simulink model "TosAeb"  (R2021a)
settings: Solver=FixedStepDiscrete  StartTime=0.0  StopTime=inf  FixedStep=0.01  RelTol=1e-3
undefined symbols (not defined in the file or in its folder): ACCModSts, AccSts1, AEB_ObjectMovingOrientation, AEB_ObjectMovingState, CN_BTCActive, CN_CurrentGearR, CN_CurrentGearR_DHT, CN_PTCActive, CN_VDCActive, CN_VSC_EspOff, CN_VSC_FCW_AEB_Error, CN_VSC_FCW_AEB_NoDispay, CN_VSC_FCW_AEB_PerfmDgdtn, CN_VSC_FCW_AEB_TempUnAvlbl, CN_VSC_VehStandstill, CNaccelAvoidMax, CNaccelAvoidMin, CNbrakePedalPress, CNcomfortStrLeftAccelMax, CNcomfortStrLeftAccelMin, CNcomfortStrRightAccelMax, CNcomfortStrRightAccelMin, CNDrvDoorOpen, CNDrvModeEco, CNDrvModeNorm, CNDrvModeOther, CNDrvModeSport, CNDrvSBRreminder, CNNull, CNobjInvalidID, CNPassDoorOpen, CNPassSBRreminder, CNPEBSAccelNegInf, CNPEBSAccelNull, CNPEBSTimeInf, CNPEBSTimeNull, CNSTOPMAX, CNSTOPNULL, CNsubjWidth, CNSwtOFF, CNSwtON, CNTTCMAX, CNTTCNULL, CNVehConfigAEBCycNone, CNVehConfigAEBNone, CNVehConfigAEBPedNone, CNVehConfigFCWNone, CNVehConfigJANone, CollisionSeverity, FALSE, FCWSnvty, JACycObjCalcInfoDefaultParams, JAPedObjCalcInfoDefaultParams, JAVehObjCalcInfoDefaultParams, latCycObjCalcInfoDefaultParams, latPedObjCalcInfoDefaultParams, longObjCalcInfoDefaultParams, ModSts, movingStatus, ObjectType ... (+1112)
libraries: simulink x3031, AEBSFunctionLib x50, Ta_Library x33, AEBSStateMachineLib x23, Mylibrary x12, simulink_extras x7, ObjSelect_lib x3, dspstat3 x2
(part 26 of 102)

== system TosAeb/FcwAebJa_Step_sys/ALG_AEBSystem/ALG_AEBController/AEB_FuncImpManagementApp/FuncBlockTimeCalc/tBlockTimeBrkReadyCalc/blockTimeTriggerCondCalc ==
  AEBModStsFuncImpArb : Inport  OutDataTypeStr=Bus: BusAEBModStsFuncImpArb
  Bus Selector : BusSelector  OutputSignals=brkReadyFuncImpArb.JA_pedPrefillFuncImpArb,brkReadyFuncImpArb.prefillFuncImpArb,brkReadyFuncImpArb.pedPrefillFuncImpArb,brkReadyFuncImpArb.cycPrefillFuncImpArb
  Bus Selector1 : BusSelector  OutputSignals=brkReadyFuncImpArb.cycPrefillFuncImpArb,brkReadyFuncImpArb.prefillFuncImpArb,brkReadyFuncImpArb.pedPrefillFuncImpArb
  Bus Selector10 : BusSelector  OutputSignals=brkReadyFuncImpArb.FCTB_ABAFuncImpArb,brkReadyFuncImpArb.ABAFuncImpArb,brkReadyFuncImpArb.pedABAFuncImpArb
  Bus Selector2 : BusSelector  OutputSignals=brkReadyFuncImpArb.pedPrefillFuncImpArb,brkReadyFuncImpArb.prefillFuncImpArb
  Bus Selector3 : BusSelector  OutputSignals=brkReadyFuncImpArb.prefillFuncImpArb
  Bus Selector4 : BusSelector  OutputSignals=brkReadyFuncImpArb.ABAFuncImpArb,brkReadyFuncImpArb.pedABAFuncImpArb
  Bus Selector5 : BusSelector  OutputSignals=brkReadyFuncImpArb.pedABAFuncImpArb
  Bus Selector6 : BusSelector  OutputSignals=brkReadyFuncImpArb.JA_cycPrefillFuncImpArb,brkReadyFuncImpArb.JA_pedPrefillFuncImpArb,brkReadyFuncImpArb.prefillFuncImpArb,brkReadyFuncImpArb.pedPrefillFuncImpArb,brkReadyFuncImpArb.cycPrefillFuncImpArb
  Bus Selector7 : BusSelector  OutputSignals=brkReadyFuncImpArb.JA_PTW_PrefillFuncImpArb,brkReadyFuncImpArb.JA_cycPrefillFuncImpArb,brkReadyFuncImpArb.JA_pedPrefillFuncImpArb,brkReadyFuncImpArb.prefillFuncImpArb,brkReadyFuncImpArb.pedPrefillFuncImpArb,brkReadyFuncImpArb.cycPrefillFuncImpArb
  Bus Selector8 : BusSelector  OutputSignals=brkReadyFuncImpArb.JA_vehPrefillFuncImpArb,brkReadyFuncImpArb.JA_PTW_PrefillFuncImpArb,brkReadyFuncImpArb.JA_cycPrefillFuncImpArb,brkReadyFuncImpArb.JA_pedPrefillFuncImpArb,brkReadyFuncImpArb.prefillFuncImpArb,brkReadyFuncImpArb.pedPrefillFuncImpArb,brkReadyFuncImpArb.cycPrefillFuncImpArb
  Bus Selector9 : BusSelector  OutputSignals=brkReadyFuncImpArb.FCTB_PrefillFuncImpArb,brkReadyFuncImpArb.JA_vehPrefillFuncImpArb,brkReadyFuncImpArb.JA_PTW_PrefillFuncImpArb,brkReadyFuncImpArb.JA_cycPrefillFuncImpArb,brkReadyFuncImpArb.JA_pedPrefillFuncImpArb,brkReadyFuncImpArb.prefillFuncImpArb,brkReadyFuncImpArb.pedPrefillFuncImpArb,brkReadyFuncImpArb.cycPrefillFuncImpArb
  Compare To Constant{,5,8,13,15..16,19,25,32,40,51} (11 alike) : simulink/Logic and Bit Operations/Compare To Constant  relop===  const=ModSts.ActiveState  OutDataTypeStr=boolean
  Compare To Constant{1..4,6..7,9..12,14,17..18,20..24,26..31,33..39,41..50,52} (42 alike) : simulink/Logic and Bit Operations/Compare To Constant  relop=~=  const=ModSts.ActiveState  OutDataTypeStr=boolean
  Logical Operator : Logic  Inputs=5  AllPortsSameDT=off  OutDataTypeStr=boolean
  Logical Operator1 : Logic  Inputs=4  AllPortsSameDT=off  OutDataTypeStr=boolean
  Logical Operator10 : Logic  Inputs=9  AllPortsSameDT=off  OutDataTypeStr=boolean
  Logical Operator11 : Logic  Inputs=4  AllPortsSameDT=off  OutDataTypeStr=boolean
  Logical Operator2 : Logic  Inputs=3  AllPortsSameDT=off  OutDataTypeStr=boolean
  Logical Operator3 : Logic  AllPortsSameDT=off  OutDataTypeStr=boolean
  Logical Operator4 : Logic  Inputs=3  AllPortsSameDT=off  OutDataTypeStr=boolean
  Logical Operator5 : Logic  AllPortsSameDT=off  OutDataTypeStr=boolean
  Logical Operator6 : Logic  Operator=OR  Inputs=11  AllPortsSameDT=off  OutDataTypeStr=boolean
  Logical Operator7 : Logic  Inputs=6  AllPortsSameDT=off  OutDataTypeStr=boolean
  Logical Operator8 : Logic  Inputs=7  AllPortsSameDT=off  OutDataTypeStr=boolean
  Logical Operator9 : Logic  Inputs=8  AllPortsSameDT=off  OutDataTypeStr=boolean
  Unit Delay{,1..10} (11 alike) : UnitDelay  InitialCondition=ModSts.OFFState  SampleTime=-1  HasFrameUpgradeWarning=on
  blockTimeTriggerCond : Outport
  wires:
  Logical Operator11/1 -> Logical Operator6/11
  Logical Operator4/1 -> Logical Operator6/10
  Logical Operator5/1 -> Logical Operator6/9
  Logical Operator10/1 -> Logical Operator6/8
  Logical Operator9/1 -> Logical Operator6/7
  Logical Operator8/1 -> Logical Operator6/6
  Logical Operator7/1 -> Logical Operator6/5
  Logical Operator/1 -> Logical Operator6/4
  Logical Operator1/1 -> Logical Operator6/3
  Logical Operator2/1 -> Logical Operator6/2
  Logical Operator3/1 -> Logical Operator6/1
  Compare To Constant52/1 -> Logical Operator11/3
  Bus Selector10/2 -> Compare To Constant52/1  "<ABAFuncImpArb>"
  Compare To Constant51/1 -> Logical Operator11/2
  Bus Selector10/3 -> Compare To Constant50/1  "<pedABAFuncImpArb>"
  Unit Delay10/1 -> Compare To Constant51/1
  Compare To Constant50/1 -> Logical Operator11/4
  Bus Selector10/1 -> Compare To Constant49/1, Unit Delay10/1  "<FCTB_ABAFuncImpArb>"
  Compare To Constant49/1 -> Logical Operator11/1
  AEBModStsFuncImpArb/1 -> Bus Selector3/1, Bus Selector2/1, Bus Selector1/1, Bus Selector/1, Bus Selector6/1, Bus Selector9/1, Bus Selector5/1, Bus Selector4/1, Bus Selector10/1, Bus Selector8/1, Bus Selector7/1
  Compare To Constant48/1 -> Logical Operator10/3
  Bus Selector9/2 -> Compare To Constant48/1  "<JA_vehPrefillFuncImpArb>"
  Compare To Constant46/1 -> Logical Operator10/5
  Bus Selector9/4 -> Compare To Constant46/1  "<JA_cycPrefillFuncImpArb>"
  Compare To Constant41/1 -> Logical Operator10/1
  Compare To Constant44/1 -> Logical Operator10/9
  Compare To Constant43/1 -> Logical Operator10/8
  Compare To Constant42/1 -> Logical Operator10/7
  Unit Delay9/1 -> Compare To Constant40/1
  Bus Selector9/1 -> Unit Delay9/1, Compare To Constant41/1  "<FCTB_PrefillFuncImpArb>"
  Bus Selector9/8 -> Compare To Constant44/1  "<cycPrefillFuncImpArb>"
  Bus Selector9/6 -> Compare To Constant42/1  "<prefillFuncImpArb>"
  Bus Selector9/7 -> Compare To Constant43/1  "<pedPrefillFuncImpArb>"
  Compare To Constant40/1 -> Logical Operator10/2
  Bus Selector9/5 -> Compare To Constant45/1  "<JA_pedPrefillFuncImpArb>"
  Compare To Constant45/1 -> Logical Operator10/6
  Bus Selector9/3 -> Compare To Constant47/1  "<JA_PTW_PrefillFuncImpArb>"
  Compare To Constant47/1 -> Logical Operator10/4
  Compare To Constant39/1 -> Logical Operator9/3
  Bus Selector8/2 -> Compare To Constant39/1  "<JA_PTW_PrefillFuncImpArb>"
  Compare To Constant37/1 -> Logical Operator9/5
  Bus Selector8/4 -> Compare To Constant37/1  "<JA_pedPrefillFuncImpArb>"
  Compare To Constant32/1 -> Logical Operator9/2
  Bus Selector8/6 -> Compare To Constant35/1  "<pedPrefillFuncImpArb>"
  Bus Selector8/5 -> Compare To Constant34/1  "<prefillFuncImpArb>"
  Bus Selector8/7 -> Compare To Constant36/1  "<cycPrefillFuncImpArb>"
  Bus Selector8/1 -> Unit Delay8/1, Compare To Constant33/1  "<JA_vehPrefillFuncImpArb>"
  Unit Delay8/1 -> Compare To Constant32/1
  Compare To Constant34/1 -> Logical Operator9/6
  Compare To Constant35/1 -> Logical Operator9/7
  Compare To Constant36/1 -> Logical Operator9/8
  Compare To Constant33/1 -> Logical Operator9/1
  Bus Selector8/3 -> Compare To Constant38/1  "<JA_cycPrefillFuncImpArb>"
  Compare To Constant38/1 -> Logical Operator9/4
  Compare To Constant31/1 -> Logical Operator8/3
  Bus Selector7/2 -> Compare To Constant31/1  "<JA_cycPrefillFuncImpArb>"
  Compare To Constant26/1 -> Logical Operator8/1
  Compare To Constant29/1 -> Logical Operator8/7
  Compare To Constant28/1 -> Logical Operator8/6
  Compare To Constant27/1 -> Logical Operator8/5
  Unit Delay7/1 -> Compare To Constant25/1
  Bus Selector7/1 -> Compare To Constant26/1, Unit Delay7/1  "<JA_PTW_PrefillFuncImpArb>"
  Bus Selector7/6 -> Compare To Constant29/1  "<cycPrefillFuncImpArb>"
  Bus Selector7/4 -> Compare To Constant27/1  "<prefillFuncImpArb>"
  Bus Selector7/5 -> Compare To Constant28/1  "<pedPrefillFuncImpArb>"
  Compare To Constant25/1 -> Logical Operator8/2
  Bus Selector7/3 -> Compare To Constant30/1  "<JA_pedPrefillFuncImpArb>"
  Compare To Constant30/1 -> Logical Operator8/4
  Compare To Constant24/1 -> Logical Operator7/3
  Bus Selector6/2 -> Compare To Constant24/1  "<JA_pedPrefillFuncImpArb>"
  Compare To Constant19/1 -> Logical Operator7/2
  Bus Selector6/4 -> Compare To Constant22/1  "<pedPrefillFuncImpArb>"
  Bus Selector6/3 -> Compare To Constant21/1  "<prefillFuncImpArb>"
  Bus Selector6/5 -> Compare To Constant23/1  "<cycPrefillFuncImpArb>"
  Bus Selector6/1 -> Unit Delay6/1, Compare To Constant20/1  "<JA_cycPrefillFuncImpArb>"
  Unit Delay6/1 -> Compare To Constant19/1
  Compare To Constant20/1 -> Logical Operator7/1
  Compare To Constant21/1 -> Logical Operator7/4
  Compare To Constant22/1 -> Logical Operator7/5
  Compare To Constant23/1 -> Logical Operator7/6
  Compare To Constant18/1 -> Logical Operator3/1
  Compare To Constant17/1 -> Logical Operator5/1
  Compare To Constant12/1 -> Logical Operator4/1
  Bus Selector4/1 -> Compare To Constant12/1, Unit Delay4/1  "<ABAFuncImpArb>"
  Compare To Constant14/1 -> Logical Operator4/3
  Unit Delay4/1 -> Compare To Constant15/1
  Bus Selector4/2 -> Compare To Constant14/1  "<pedABAFuncImpArb>"
  Compare To Constant15/1 -> Logical Operator4/2
  Compare To Constant5/1 -> Logical Operator1/2
  Bus Selector1/1 -> Compare To Constant9/1, Unit Delay1/1  "<cycPrefillFuncImpArb>"
  Unit Delay1/1 -> Compare To Constant5/1
  Compare To Constant6/1 -> Logical Operator1/3
  Bus Selector3/1 -> Compare To Constant18/1, Unit Delay3/1  "<prefillFuncImpArb>"
  Compare To Constant13/1 -> Logical Operator3/2
  Unit Delay3/1 -> Compare To Constant13/1
  Logical Operator6/1 -> blockTimeTriggerCond/1
  Unit Delay2/1 -> Compare To Constant8/1
  Bus Selector2/1 -> Compare To Constant10/1, Unit Delay2/1  "<pedPrefillFuncImpArb>"
  Compare To Constant16/1 -> Logical Operator5/2
  Unit Delay5/1 -> Compare To Constant16/1
  Bus Selector5/1 -> Compare To Constant17/1, Unit Delay5/1  "<pedABAFuncImpArb>"
  Compare To Constant11/1 -> Logical Operator2/3
  Bus Selector1/2 -> Compare To Constant6/1  "<prefillFuncImpArb>"
  Compare To Constant9/1 -> Logical Operator1/1
  Compare To Constant7/1 -> Logical Operator1/4
  Bus Selector1/3 -> Compare To Constant7/1  "<pedPrefillFuncImpArb>"
  Compare To Constant10/1 -> Logical Operator2/1
  Compare To Constant4/1 -> Logical Operator/5
  Compare To Constant3/1 -> Logical Operator/4
  Compare To Constant2/1 -> Logical Operator/3
  Compare To Constant8/1 -> Logical Operator2/2
  Bus Selector2/2 -> Compare To Constant11/1  "<prefillFuncImpArb>"
  Compare To Constant1/1 -> Logical Operator/1
  Unit Delay/1 -> Compare To Constant/1
  Bus Selector/1 -> Unit Delay/1, Compare To Constant1/1  "<JA_pedPrefillFuncImpArb>"
  Bus Selector/4 -> Compare To Constant4/1  "<cycPrefillFuncImpArb>"
  Bus Selector/2 -> Compare To Constant2/1  "<prefillFuncImpArb>"
  Bus Selector/3 -> Compare To Constant3/1  "<pedPrefillFuncImpArb>"
  Compare To Constant/1 -> Logical Operator/2

== system TosAeb/FcwAebJa_Step_sys/ALG_AEBSystem/ALG_AEBController/AEB_FuncImpManagementApp/FuncBlockTimeCalc/tBlockTimeWarningCalc/BlockTimeCalc ==
  blockTimeTriggerCond : Inport
  BlockTimeCalc : Stateflow chart  TreatAsAtomicUnit=on
  Constant : Constant  Value=Ts  OutDataTypeStr=single
  Constant1 : Constant  Value=P_AEB_FIM_WarningBlockTimeThres  OutDataTypeStr=single
  blockTime : Outport
  wires:
  BlockTimeCalc/1(blockTime) -> blockTime/1
  blockTimeTriggerCond/1 -> BlockTimeCalc/1(blockTimeTriggerCond)
  Constant/1 -> BlockTimeCalc/2(Ts)
  Constant1/1 -> BlockTimeCalc/3(PblockTime)

== system TosAeb/FcwAebJa_Step_sys/ALG_AEBSystem/ALG_AEBController/AEB_FuncImpManagementApp/FuncBlockTimeCalc/tBlockTimeWarningCalc/blockTimeTriggerCondCalc ==
  AEBModStsFuncImpArb : Inport  OutDataTypeStr=Bus: BusAEBModStsFuncImpArb
  Bus Selector1 : BusSelector  OutputSignals=warningFuncImpArb.preWarningFuncImpArb,warningFuncImpArb.pedPreWarningFuncImpArb,warningFuncImpArb.acuteWarningFuncImpArb
  Bus Selector2 : BusSelector  OutputSignals=warningFuncImpArb.pedPreWarningFuncImpArb,warningFuncImpArb.acuteWarningFuncImpArb
  Bus Selector5 : BusSelector  OutputSignals=warningFuncImpArb.acuteWarningFuncImpArb
  Compare To Constant{6..7,9..11,17} (6 alike) : simulink/Logic and Bit Operations/Compare To Constant  relop=~=  const=ModSts.ActiveState  OutDataTypeStr=boolean
  Compare To Constant16 : simulink/Logic and Bit Operations/Compare To Constant  relop===  const=ModSts.ActiveState  OutDataTypeStr=boolean
  Compare To Constant5 : simulink/Logic and Bit Operations/Compare To Constant  relop===  const=ModSts.ActiveState  OutDataTypeStr=boolean
  Compare To Constant8 : simulink/Logic and Bit Operations/Compare To Constant  relop===  const=ModSts.ActiveState  OutDataTypeStr=boolean
  Logical Operator1 : Logic  Inputs=4  AllPortsSameDT=off  OutDataTypeStr=boolean
  Logical Operator2 : Logic  Inputs=3  AllPortsSameDT=off  OutDataTypeStr=boolean
  Logical Operator5 : Logic  AllPortsSameDT=off  OutDataTypeStr=boolean
  Logical Operator6 : Logic  Operator=OR  Inputs=3  AllPortsSameDT=off  OutDataTypeStr=boolean
  Unit Delay1 : UnitDelay  InitialCondition=ModSts.OFFState  SampleTime=-1  HasFrameUpgradeWarning=on
  Unit Delay2 : UnitDelay  InitialCondition=ModSts.OFFState  SampleTime=-1  HasFrameUpgradeWarning=on
  Unit Delay5 : UnitDelay  InitialCondition=ModSts.OFFState  SampleTime=-1  HasFrameUpgradeWarning=on
  blockTimeTriggerCond : Outport
  wires:
  Logical Operator5/1 -> Logical Operator6/1
  AEBModStsFuncImpArb/1 -> Bus Selector1/1, Bus Selector2/1, Bus Selector5/1
  Bus Selector2/2 -> Compare To Constant11/1  "<acuteWarningFuncImpArb>"
  Compare To Constant8/1 -> Logical Operator2/2
  Compare To Constant10/1 -> Logical Operator2/1
  Bus Selector1/3 -> Compare To Constant7/1  "<acuteWarningFuncImpArb>"
  Compare To Constant7/1 -> Logical Operator1/4
  Compare To Constant9/1 -> Logical Operator1/1
  Bus Selector1/2 -> Compare To Constant6/1  "<pedPreWarningFuncImpArb>"
  Compare To Constant11/1 -> Logical Operator2/3
  Bus Selector5/1 -> Unit Delay5/1, Compare To Constant17/1  "<acuteWarningFuncImpArb>"
  Unit Delay5/1 -> Compare To Constant16/1
  Compare To Constant16/1 -> Logical Operator5/2
  Bus Selector2/1 -> Unit Delay2/1, Compare To Constant10/1  "<pedPreWarningFuncImpArb>"
  Unit Delay2/1 -> Compare To Constant8/1
  Logical Operator6/1 -> blockTimeTriggerCond/1
  Compare To Constant6/1 -> Logical Operator1/3
  Unit Delay1/1 -> Compare To Constant5/1
  Bus Selector1/1 -> Unit Delay1/1, Compare To Constant9/1  "<preWarningFuncImpArb>"
  Compare To Constant5/1 -> Logical Operator1/2
  Logical Operator1/1 -> Logical Operator6/3
  Logical Operator2/1 -> Logical Operator6/2
  Compare To Constant17/1 -> Logical Operator5/1

== system TosAeb/FcwAebJa_Step_sys/ALG_AEBSystem/ALG_AEBController/AEB_FuncImpManagementApp/FuncImpArbCalc/BrkFuncImpArbCalc/BrkFumcActiveCondCalc ==
  AEBpartFuncImpArb : Inport
  cycAEBpartFuncImpArb : Inport  Port=2
  AEBfullFuncImpArb : Inport  Port=3
  JA_vehAEBfullFuncImpArb : Inport  Port=4
  cycAEBfullFuncImpArb : Inport  Port=5
  pedAEBfullFuncImpArb : Inport  Port=6
  EBAFuncImpArb : Inport  Port=7
  FCTBFuncImpArb : Inport  Port=8
  JA_PTW_AEBFuncImpArb : Inport  Port=9
  JA_cycAEBFuncImpArb : Inport  Port=10
  JA_pedAEBFuncImpArb : Inport  Port=11
  Compare To Constant{,1..10} (11 alike) : simulink/Logic and Bit Operations/Compare To Constant  relop===  const=ModSts.ActiveState  OutDataTypeStr=boolean
  Logical Operator : Logic  Operator=OR  Inputs=11  AllPortsSameDT=off  OutDataTypeStr=boolean
  brkFuncActvCond : Outport
  wires:
  Compare To Constant10/1 -> Logical Operator/11
  Compare To Constant9/1 -> Logical Operator/10
  Compare To Constant8/1 -> Logical Operator/9
  JA_pedAEBFuncImpArb/1 -> Compare To Constant10/1
  JA_cycAEBFuncImpArb/1 -> Compare To Constant9/1
  JA_PTW_AEBFuncImpArb/1 -> Compare To Constant8/1
  Logical Operator/1 -> brkFuncActvCond/1
  Compare To Constant7/1 -> Logical Operator/8
  Compare To Constant/1 -> Logical Operator/1
  Compare To Constant6/1 -> Logical Operator/7
  Compare To Constant1/1 -> Logical Operator/2
  Compare To Constant5/1 -> Logical Operator/6
  Compare To Constant2/1 -> Logical Operator/3
  Compare To Constant4/1 -> Logical Operator/5
  Compare To Constant3/1 -> Logical Operator/4
  cycAEBfullFuncImpArb/1 -> Compare To Constant4/1
  pedAEBfullFuncImpArb/1 -> Compare To Constant5/1
  FCTBFuncImpArb/1 -> Compare To Constant7/1
  EBAFuncImpArb/1 -> Compare To Constant6/1
  AEBfullFuncImpArb/1 -> Compare To Constant2/1
  JA_vehAEBfullFuncImpArb/1 -> Compare To Constant3/1
  cycAEBpartFuncImpArb/1 -> Compare To Constant1/1
  AEBpartFuncImpArb/1 -> Compare To Constant/1

== system TosAeb/FcwAebJa_Step_sys/ALG_AEBSystem/ALG_AEBController/AEB_FuncImpManagementApp/FuncImpArbCalc/BrkFuncImpArbCalc/BrkFuncImpAborCondCalc ==
  AEBpartModStsArb : Inport
  AEBfullModStsArb : Inport  Port=2
  EBAModStsArb : Inport  Port=3
  cycAEBpartModStsArb : Inport  Port=4
  cycAEBfullModStsArb : Inport  Port=5
  pedAEBfullModStsArb : Inport  Port=6
  JA_vehAEBModStsArb : Inport  Port=7
  JA_PTW_AEBModStsArb : Inport  Port=8
  JA_cycAEBModStsArb : Inport  Port=9
  JA_pedAEBModStsArb : Inport  Port=10
  AEBfullFuncImpAborCondCalc : Subsystem
  AEBpartFuncImpAborCondCalc : Subsystem
  Bus Creator : BusCreator  Inputs=10
  Bus Selector : BusSelector  OutputSignals=AEBpartModStsArb,AEBfullModStsArb,EBAModStsArb,cycAEBpartModStsArb,cycAEBfullModStsArb,pedAEBfullModStsArb,JA_vehAEBModStsArb,JA_PTW_AEBModStsArb,JA_cycAEBModStsArb,JA_pedAEBModStsArb
  Bus Selector1 : BusSelector  OutputSignals=AEBfullModStsArb,pedAEBfullModStsArb,cycAEBpartModStsArb,cycAEBfullModStsArb,JA_vehAEBModStsArb,EBAModStsArb,JA_PTW_AEBModStsArb,JA_cycAEBModStsArb,JA_pedAEBModStsArb
  Bus Selector2 : BusSelector  OutputSignals=AEBfullModStsArb,pedAEBfullModStsArb,cycAEBfullModStsArb,JA_vehAEBModStsArb,EBAModStsArb,JA_PTW_AEBModStsArb,JA_cycAEBModStsArb,JA_pedAEBModStsArb
  Bus Selector3 : BusSelector  OutputSignals=AEBfullModStsArb,pedAEBfullModStsArb,cycAEBfullModStsArb,EBAModStsArb,JA_PTW_AEBModStsArb,JA_cycAEBModStsArb,JA_pedAEBModStsArb
  Bus Selector4 : BusSelector  OutputSignals=pedAEBfullModStsArb,cycAEBfullModStsArb,EBAModStsArb,JA_PTW_AEBModStsArb,JA_cycAEBModStsArb,JA_pedAEBModStsArb
  Bus Selector5 : BusSelector  OutputSignals=pedAEBfullModStsArb,EBAModStsArb
  Bus Selector6 : BusSelector  OutputSignals=EBAModStsArb
  Bus Selector7 : BusSelector  OutputSignals=pedAEBfullModStsArb,cycAEBfullModStsArb,EBAModStsArb
  Bus Selector8 : BusSelector  OutputSignals=pedAEBfullModStsArb,cycAEBfullModStsArb,EBAModStsArb,JA_pedAEBModStsArb
  Bus Selector9 : BusSelector  OutputSignals=JA_cycAEBModStsArb,pedAEBfullModStsArb,JA_pedAEBModStsArb,cycAEBfullModStsArb,EBAModStsArb
  EBAFuncImpAborCondCalc : Subsystem
  FCTBFuncImpAborCondCalc : Subsystem
  JA_PTW_AEBfullFuncImpAborCondCalc : Subsystem
  JA_cycAEBfullFuncImpAborCondCalc : Subsystem
  JA_pedAEBfullFuncImpAborCondCalc : Subsystem
  JA_vehAEBfullFuncImpAborCondCalc : Subsystem
  cycAEBfullFuncImpAborCondCalc : Subsystem
  cycAEBpartFuncImpAborCondCalc : Subsystem
  pedAEBfullFuncImpAborCondCalc : Subsystem
  FCTBFuncImpAborCond : Outport
  AEBpartFuncImpAborCond : Outport  Port=2
  cycAEBpartFuncImpAborCond : Outport  Port=3
  JA_vehAEBfullFuncImpAborCond : Outport  Port=4
  AEBfullFuncImpAborCond : Outport  Port=5
  cycAEBfullFuncImpAborCond : Outport  Port=6
  pedAEBfullFuncImpAborCond : Outport  Port=7
  EBAFuncImpAborCond : Outport  Port=8
  JA_pedAEBfullFuncImpAborCond : Outport  Port=9
  JA_cycAEBfullFuncImpAborCond : Outport  Port=10
  JA_PTW_AEBfullFuncImpAborCond : Outport  Port=11
  wires:
  Bus Selector4/5 -> AEBfullFuncImpAborCondCalc/5(JA_cycAEBfullModStsArb)  "<JA_cycAEBModStsArb>"
  Bus Selector/3 -> FCTBFuncImpAborCondCalc/3(EBAModStsArb)  "<EBAModStsArb>"
  Bus Selector9/4 -> JA_PTW_AEBfullFuncImpAborCondCalc/4(cycAEBfullModStsArb)  "<cycAEBfullModStsArb>"
  Bus Selector1/3 -> AEBpartFuncImpAborCondCalc/3(cycAEBpartModStsArb)  "<cycAEBpartModStsArb>"
  JA_cycAEBfullFuncImpAborCondCalc/1(JA_cycAEBfullFuncImpAborCond) -> JA_cycAEBfullFuncImpAborCond/1
  Bus Selector8/1 -> JA_cycAEBfullFuncImpAborCondCalc/1(cycAEBfullModStsArb)  "<pedAEBfullModStsArb>"
  JA_vehAEBModStsArb/1 -> Bus Creator/7  "JA_vehAEBModStsArb"
  Bus Selector7/1 -> JA_pedAEBfullFuncImpAborCondCalc/1(cycAEBfullModStsArb)  "<pedAEBfullModStsArb>"
  Bus Selector1/7 -> AEBpartFuncImpAborCondCalc/7(JA_PTW_AEBfullModStsArb)  "<JA_PTW_AEBModStsArb>"
  JA_PTW_AEBModStsArb/1 -> Bus Creator/8  "JA_PTW_AEBModStsArb"
  Bus Selector1/1 -> AEBpartFuncImpAborCondCalc/1(AEBfullModStsArb)  "<AEBfullModStsArb>"
  Bus Selector2/5 -> cycAEBpartFuncImpAborCondCalc/5(EBAModStsArb)  "<EBAModStsArb>"
  JA_pedAEBModStsArb/1 -> Bus Creator/10  "JA_pedAEBModStsArb"
  Bus Selector8/4 -> JA_cycAEBfullFuncImpAborCondCalc/4(JA_pedAEBModStsArb)  "<JA_pedAEBModStsArb>"
  Bus Selector6/1 -> pedAEBfullFuncImpAborCondCalc/1(EBAModStsArb)  "<EBAModStsArb>"
  Bus Selector2/8 -> cycAEBpartFuncImpAborCondCalc/8(JA_pedAEBfullModStsArb)  "<JA_pedAEBModStsArb>"
  Bus Selector1/8 -> AEBpartFuncImpAborCondCalc/8(JA_cycAEBfullModStsArb)  "<JA_cycAEBModStsArb>"
  Bus Selector1/6 -> AEBpartFuncImpAborCondCalc/6(EBAModStsArb)  "<EBAModStsArb>"
  AEBfullModStsArb/1 -> Bus Creator/2  "AEBfullModStsArb"
  pedAEBfullModStsArb/1 -> Bus Creator/6  "pedAEBfullModStsArb"
  Bus Selector2/6 -> cycAEBpartFuncImpAborCondCalc/6(JA_PTW_AEBfullModStsArb)  "<JA_PTW_AEBModStsArb>"
  AEBfullFuncImpAborCondCalc/1(AEBfullFuncImpAborCond) -> AEBfullFuncImpAborCond/1
  Bus Selector/6 -> FCTBFuncImpAborCondCalc/6(cycAEBpartModStsArb)  "<pedAEBfullModStsArb>"
  AEBpartModStsArb/1 -> Bus Creator/1  "AEBpartModStsArb"
  cycAEBfullFuncImpAborCondCalc/1(cycAEBfullFuncImpAborCond) -> cycAEBfullFuncImpAborCond/1
  Bus Selector3/1 -> JA_vehAEBfullFuncImpAborCondCalc/1(AEBfullModStsArb)  "<AEBfullModStsArb>"
  Bus Selector4/4 -> AEBfullFuncImpAborCondCalc/4(JA_PTW_AEBfullModStsArb)  "<JA_PTW_AEBModStsArb>"
  Bus Selector3/5 -> JA_vehAEBfullFuncImpAborCondCalc/5(JA_PTW_AEBModStsArb)  "<JA_PTW_AEBModStsArb>"
  Bus Selector4/6 -> AEBfullFuncImpAborCondCalc/6(JA_pedAEBfullModStsArb)  "<JA_pedAEBModStsArb>"
  Bus Selector2/2 -> cycAEBpartFuncImpAborCondCalc/2(pedAEBfullModStsArb)  "<pedAEBfullModStsArb>"
  cycAEBpartFuncImpAborCondCalc/1(cycAEBpartFuncImpAborCond) -> cycAEBpartFuncImpAborCond/1
  Bus Selector9/3 -> JA_PTW_AEBfullFuncImpAborCondCalc/3(JA_pedAEBfullModStsArb)  "<JA_pedAEBModStsArb>"
  EBAModStsArb/1 -> Bus Creator/3  "EBAModStsArb"
  FCTBFuncImpAborCondCalc/1(FCTBFuncImpAborCond) -> FCTBFuncImpAborCond/1
  Bus Selector/1 -> FCTBFuncImpAborCondCalc/1(AEBpartModStsArb)  "<AEBpartModStsArb>"
  Bus Selector8/2 -> JA_cycAEBfullFuncImpAborCondCalc/2(pedAEBfullModStsArb)  "<cycAEBfullModStsArb>"
  Bus Selector2/3 -> cycAEBpartFuncImpAborCondCalc/3(cycAEBfullModStsArb)  "<cycAEBfullModStsArb>"
  Bus Creator/1 -> Bus Selector/1, Bus Selector1/1, Bus Selector2/1, Bus Selector3/1, Bus Selector4/1, Bus Selector9/1, Bus Selector8/1, Bus Selector7/1, Bus Selector5/1, Bus Selector6/1
  Bus Selector9/1 -> JA_PTW_AEBfullFuncImpAborCondCalc/1(AEBfullModStsArb)  "<JA_cycAEBModStsArb>"
  Bus Selector3/6 -> JA_vehAEBfullFuncImpAborCondCalc/6(JA_cycAEBModStsArb)  "<JA_cycAEBModStsArb>"
  Bus Selector2/1 -> cycAEBpartFuncImpAborCondCalc/1(AEBfullModStsArb)  "<AEBfullModStsArb>"
  AEBpartFuncImpAborCondCalc/1(AEBpartFuncImpAborCond) -> AEBpartFuncImpAborCond/1
  Bus Selector3/3 -> JA_vehAEBfullFuncImpAborCondCalc/3(pedAEBfullModStsArb)  "<cycAEBfullModStsArb>"
  Bus Selector1/9 -> AEBpartFuncImpAborCondCalc/9(JA_pedAEBfullModStsArb)  "<JA_pedAEBModStsArb>"
  Bus Selector2/7 -> cycAEBpartFuncImpAborCondCalc/7(JA_cycAEBfullModStsArb)  "<JA_cycAEBModStsArb>"
  Bus Selector7/3 -> JA_pedAEBfullFuncImpAborCondCalc/3(EBAModStsArb)  "<EBAModStsArb>"
  Bus Selector3/2 -> JA_vehAEBfullFuncImpAborCondCalc/2(cycAEBfullModStsArb)  "<pedAEBfullModStsArb>"
  Bus Selector3/7 -> JA_vehAEBfullFuncImpAborCondCalc/7(JA_pedAEBModStsArb)  "<JA_pedAEBModStsArb>"
  JA_vehAEBfullFuncImpAborCondCalc/1(JA_vehAEBfullFuncImpAborCond) -> JA_vehAEBfullFuncImpAborCond/1
  Bus Selector1/4 -> AEBpartFuncImpAborCondCalc/4(cycAEBfullModStsArb)  "<cycAEBfullModStsArb>"
  EBAFuncImpAborCondCalc/1(EBAFuncImpAborCond) -> EBAFuncImpAborCond/1
  cycAEBpartModStsArb/1 -> Bus Creator/4  "cycAEBpartModStsArb"
  JA_cycAEBModStsArb/1 -> Bus Creator/9  "JA_cycAEBModStsArb"
  Bus Selector5/1 -> cycAEBfullFuncImpAborCondCalc/1(pedAEBfullModStsArb)  "<pedAEBfullModStsArb>"
  Bus Selector9/5 -> JA_PTW_AEBfullFuncImpAborCondCalc/5(EBAModStsArb)  "<EBAModStsArb>"
  Bus Selector/7 -> FCTBFuncImpAborCondCalc/7(JA_vehAEBfullModStsArb)  "<JA_vehAEBModStsArb>"
  Bus Selector7/2 -> JA_pedAEBfullFuncImpAborCondCalc/2(pedAEBfullModStsArb)  "<cycAEBfullModStsArb>"
  pedAEBfullFuncImpAborCondCalc/1(pedAEBfullFuncImpAborCond) -> pedAEBfullFuncImpAborCond/1
  Bus Selector/9 -> FCTBFuncImpAborCondCalc/9(JA_cycAEBModStsArb)  "<JA_cycAEBModStsArb>"
  Bus Selector8/3 -> JA_cycAEBfullFuncImpAborCondCalc/3(EBAModStsArb)  "<EBAModStsArb>"
  Bus Selector5/2 -> cycAEBfullFuncImpAborCondCalc/2(EBAModStsArb)  "<EBAModStsArb>"
  Bus Selector4/2 -> AEBfullFuncImpAborCondCalc/2(cycAEBfullModStsArb)  "<cycAEBfullModStsArb>"
  Bus Selector/8 -> FCTBFuncImpAborCondCalc/8(JA_PTW_AEBModStsArb)  "<JA_PTW_AEBModStsArb>"
  Bus Selector4/1 -> AEBfullFuncImpAborCondCalc/1(pedAEBfullModStsArb)  "<pedAEBfullModStsArb>"
  Bus Selector3/4 -> JA_vehAEBfullFuncImpAborCondCalc/4(EBAModStsArb)  "<EBAModStsArb>"
  Bus Selector2/4 -> cycAEBpartFuncImpAborCondCalc/4(JA_vehAEBfullModStsArb)  "<JA_vehAEBModStsArb>"
  Bus Selector9/2 -> JA_PTW_AEBfullFuncImpAborCondCalc/2(pedAEBfullModStsArb)  "<pedAEBfullModStsArb>"
  Bus Selector/10 -> FCTBFuncImpAborCondCalc/10(JA_pedAEBModStsArb)  "<JA_pedAEBModStsArb>"
  Bus Selector4/3 -> AEBfullFuncImpAborCondCalc/3(EBAModStsArb)  "<EBAModStsArb>"
  Bus Selector/4 -> FCTBFuncImpAborCondCalc/4(pedAEBfullModStsArb)  "<cycAEBpartModStsArb>"
  Bus Selector1/5 -> AEBpartFuncImpAborCondCalc/5(JA_vehAEBfullModStsArb)  "<JA_vehAEBModStsArb>"
  JA_pedAEBfullFuncImpAborCondCalc/1(JA_pedAEBfullFuncImpAborCond) -> JA_pedAEBfullFuncImpAborCond/1
  Bus Selector/5 -> FCTBFuncImpAborCondCalc/5(cycAEBfullModStsArb)  "<cycAEBfullModStsArb>"
  Bus Selector/2 -> FCTBFuncImpAborCondCalc/2(AEBfullModStsArb)  "<AEBfullModStsArb>"
  cycAEBfullModStsArb/1 -> Bus Creator/5  "cycAEBfullModStsArb"
  JA_PTW_AEBfullFuncImpAborCondCalc/1(JA_PTW_AEBfullFuncImpAborCond) -> JA_PTW_AEBfullFuncImpAborCond/1
  Bus Selector1/2 -> AEBpartFuncImpAborCondCalc/2(pedAEBfullModStsArb)  "<pedAEBfullModStsArb>"

== system TosAeb/FcwAebJa_Step_sys/ALG_AEBSystem/ALG_AEBController/AEB_FuncImpManagementApp/FuncImpArbCalc/BrkFuncImpArbCalc/BrkFuncImpAborCondCalc1 ==
  AEBpartModStsArb : Inport
  AEBfullModStsArb : Inport  Port=2
  EBAModStsArb : Inport  Port=3
  cycAEBpartModStsArb : Inport  Port=4
  cycAEBfullModStsArb : Inport  Port=5
  pedAEBfullModStsArb : Inport  Port=6
  JA_vehAEBModStsArb : Inport  Port=7
  JA_PTW_AEBModStsArb : Inport  Port=8
  JA_cycAEBModStsArb : Inport  Port=9
  JA_pedAEBModStsArb : Inport  Port=10
  AEBfullFuncImpAborCondCalc : Subsystem
  AEBpartFuncImpAborCondCalc : Subsystem
  Bus Creator : BusCreator  Inputs=10
  Bus Selector : BusSelector  OutputSignals=AEBpartModStsArb,AEBfullModStsArb,EBAModStsArb,cycAEBpartModStsArb,cycAEBfullModStsArb,pedAEBfullModStsArb,JA_vehAEBModStsArb,JA_PTW_AEBModStsArb,JA_cycAEBModStsArb,JA_pedAEBModStsArb
  Bus Selector1 : BusSelector  OutputSignals=AEBfullModStsArb,pedAEBfullModStsArb,JA_pedAEBModStsArb,cycAEBpartModStsArb,cycAEBfullModStsArb,JA_vehAEBModStsArb,EBAModStsArb
  Bus Selector2 : BusSelector  OutputSignals=AEBfullModStsArb,pedAEBfullModStsArb,JA_pedAEBModStsArb,cycAEBfullModStsArb,JA_vehAEBModStsArb,EBAModStsArb
  Bus Selector3 : BusSelector  OutputSignals=AEBfullModStsArb,pedAEBfullModStsArb,cycAEBfullModStsArb,EBAModStsArb,JA_PTW_AEBModStsArb,JA_cycAEBModStsArb,JA_pedAEBModStsArb
  Bus Selector4 : BusSelector  OutputSignals=pedAEBfullModStsArb,cycAEBfullModStsArb,EBAModStsArb
  Bus Selector5 : BusSelector  OutputSignals=pedAEBfullModStsArb,EBAModStsArb
  Bus Selector6 : BusSelector  OutputSignals=EBAModStsArb
  Bus Selector7 : BusSelector  OutputSignals=AEBfullModStsArb,pedAEBfullModStsArb,cycAEBfullModStsArb,EBAModStsArb
  Bus Selector8 : BusSelector  OutputSignals=AEBfullModStsArb,pedAEBfullModStsArb,cycAEBfullModStsArb,EBAModStsArb,JA_pedAEBModStsArb
  Bus Selector9 : BusSelector  OutputSignals=AEBfullModStsArb,pedAEBfullModStsArb,cycAEBpartModStsArb,cycAEBfullModStsArb,JA_vehAEBModStsArb,EBAModStsArb
  EBAFuncImpAborCondCalc : Subsystem
  FCTBFuncImpAborCondCalc : Subsystem
  JA_PTW_AEBfullFuncImpAborCondCalc : Subsystem
  JA_cycAEBfullFuncImpAborCondCalc : Subsystem
  JA_pedAEBfullFuncImpAborCondCalc : Subsystem
  JA_vehAEBfullFuncImpAborCondCalc : Subsystem
  cycAEBfullFuncImpAborCondCalc : Subsystem
  cycAEBpartFuncImpAborCondCalc : Subsystem
  pedAEBfullFuncImpAborCondCalc : Subsystem
  FCTBFuncImpAborCond : Outport
  AEBpartFuncImpAborCond : Outport  Port=2
  cycAEBpartFuncImpAborCond : Outport  Port=3
  JA_vehAEBfullFuncImpAborCond : Outport  Port=4
  AEBfullFuncImpAborCond : Outport  Port=5
  cycAEBfullFuncImpAborCond : Outport  Port=6
  pedAEBfullFuncImpAborCond : Outport  Port=7
  EBAFuncImpAborCond : Outport  Port=8
  JA_pedAEBfullFuncImpAborCond : Outport  Port=9
  JA_cycAEBfullFuncImpAborCond : Outport  Port=10
  JA_PTW_AEBfullFuncImpAborCond : Outport  Port=11
  wires:
  EBAFuncImpAborCondCalc/1(EBAFuncImpAborCond) -> EBAFuncImpAborCond/1  "EBAFuncImpAbor"
  pedAEBfullFuncImpAborCondCalc/1(pedAEBfullFuncImpAborCond) -> pedAEBfullFuncImpAborCond/1  "pedAEBfullFuncImpAbor"
  cycAEBfullFuncImpAborCondCalc/1(cycAEBfullFuncImpAborCond) -> cycAEBfullFuncImpAborCond/1  "cycAEBfullFuncImpAbor"
  AEBfullFuncImpAborCondCalc/1(AEBfullFuncImpAborCond) -> AEBfullFuncImpAborCond/1  "AEBfullFuncImpAbor"
  JA_vehAEBfullFuncImpAborCondCalc/1(JA_vehAEBfullFuncImpAborCond) -> JA_vehAEBfullFuncImpAborCond/1  "JA_vehAEBfullFuncImpAbor"
  cycAEBpartFuncImpAborCondCalc/1(cycAEBpartFuncImpAborCond) -> cycAEBpartFuncImpAborCond/1  "cycAEBpartFuncImpAbor"
  AEBpartFuncImpAborCondCalc/1(AEBpartFuncImpAborCond) -> AEBpartFuncImpAborCond/1  "AEBpartFuncImpAbor"
  FCTBFuncImpAborCondCalc/1(FCTBFuncImpAborCond) -> FCTBFuncImpAborCond/1  "FCTBFuncImpAbor"
  AEBpartModStsArb/1 -> Bus Creator/1  "AEBpartModStsArb"
  AEBfullModStsArb/1 -> Bus Creator/2  "AEBfullModStsArb"
  EBAModStsArb/1 -> Bus Creator/3  "EBAModStsArb"
  cycAEBpartModStsArb/1 -> Bus Creator/4  "cycAEBpartModStsArb"
  cycAEBfullModStsArb/1 -> Bus Creator/5  "cycAEBfullModStsArb"
  pedAEBfullModStsArb/1 -> Bus Creator/6  "pedAEBfullModStsArb"
  JA_vehAEBModStsArb/1 -> Bus Creator/7  "JA_vehAEBModStsArb"
  JA_PTW_AEBModStsArb/1 -> Bus Creator/8  "JA_PTW_AEBModStsArb"
  JA_cycAEBModStsArb/1 -> Bus Creator/9  "JA_cycAEBModStsArb"
  JA_pedAEBModStsArb/1 -> Bus Creator/10  "JA_pedAEBModStsArb"
  Bus Selector/1 -> FCTBFuncImpAborCondCalc/1(AEBpartModStsArb)  "<AEBpartModStsArb>"
  Bus Selector/2 -> FCTBFuncImpAborCondCalc/2(AEBfullModStsArb)  "<AEBfullModStsArb>"
  Bus Selector/3 -> FCTBFuncImpAborCondCalc/3(EBAModStsArb)  "<EBAModStsArb>"
  Bus Selector/4 -> FCTBFuncImpAborCondCalc/4(pedAEBfullModStsArb)  "<cycAEBpartModStsArb>"
  Bus Selector/5 -> FCTBFuncImpAborCondCalc/5(cycAEBfullModStsArb)  "<cycAEBfullModStsArb>"
  Bus Selector/6 -> FCTBFuncImpAborCondCalc/6(cycAEBpartModStsArb)  "<pedAEBfullModStsArb>"
  Bus Selector/7 -> FCTBFuncImpAborCondCalc/7(JA_vehAEBfullModStsArb)  "<JA_vehAEBModStsArb>"
  Bus Selector/8 -> FCTBFuncImpAborCondCalc/8(JA_PTW_AEBModStsArb)  "<JA_PTW_AEBModStsArb>"
  Bus Selector/9 -> FCTBFuncImpAborCondCalc/9(JA_cycAEBModStsArb)  "<JA_cycAEBModStsArb>"
  Bus Selector/10 -> FCTBFuncImpAborCondCalc/10(JA_pedAEBModStsArb)  "<JA_pedAEBModStsArb>"
  Bus Selector1/1 -> AEBpartFuncImpAborCondCalc/1(AEBfullModStsArb)  "<AEBfullModStsArb>"
  Bus Selector1/2 -> AEBpartFuncImpAborCondCalc/2(pedAEBfullModStsArb)  "<pedAEBfullModStsArb>"
  Bus Selector1/4 -> AEBpartFuncImpAborCondCalc/4(cycAEBpartModStsArb)  "<cycAEBpartModStsArb>"
  Bus Selector1/5 -> AEBpartFuncImpAborCondCalc/5(cycAEBfullModStsArb)  "<cycAEBfullModStsArb>"
  Bus Selector1/6 -> AEBpartFuncImpAborCondCalc/6(JA_vehAEBfullModStsArb)  "<JA_vehAEBModStsArb>"
  Bus Selector1/7 -> AEBpartFuncImpAborCondCalc/7(EBAModStsArb)  "<EBAModStsArb>"
  Bus Selector2/1 -> cycAEBpartFuncImpAborCondCalc/1(AEBfullModStsArb)  "<AEBfullModStsArb>"
  Bus Selector2/2 -> cycAEBpartFuncImpAborCondCalc/2(pedAEBfullModStsArb)  "<pedAEBfullModStsArb>"
  Bus Selector2/4 -> cycAEBpartFuncImpAborCondCalc/4(cycAEBfullModStsArb)  "<cycAEBfullModStsArb>"
  Bus Selector2/5 -> cycAEBpartFuncImpAborCondCalc/5(JA_vehAEBfullModStsArb)  "<JA_vehAEBModStsArb>"
  Bus Selector2/6 -> cycAEBpartFuncImpAborCondCalc/6(EBAModStsArb)  "<EBAModStsArb>"
  Bus Selector3/1 -> JA_vehAEBfullFuncImpAborCondCalc/1(AEBfullModStsArb)  "<AEBfullModStsArb>"
  Bus Selector3/2 -> JA_vehAEBfullFuncImpAborCondCalc/2(cycAEBfullModStsArb)  "<pedAEBfullModStsArb>"
  Bus Selector3/3 -> JA_vehAEBfullFuncImpAborCondCalc/3(pedAEBfullModStsArb)  "<cycAEBfullModStsArb>"
  Bus Selector3/4 -> JA_vehAEBfullFuncImpAborCondCalc/4(EBAModStsArb)  "<EBAModStsArb>"
  Bus Selector4/3 -> AEBfullFuncImpAborCondCalc/3(EBAModStsArb)  "<EBAModStsArb>"
  Bus Selector4/2 -> AEBfullFuncImpAborCondCalc/2(cycAEBfullModStsArb)  "<cycAEBfullModStsArb>"
  Bus Selector4/1 -> AEBfullFuncImpAborCondCalc/1(pedAEBfullModStsArb)  "<pedAEBfullModStsArb>"
  Bus Selector5/1 -> cycAEBfullFuncImpAborCondCalc/1(pedAEBfullModStsArb)  "<pedAEBfullModStsArb>"
  Bus Selector5/2 -> cycAEBfullFuncImpAborCondCalc/2(EBAModStsArb)  "<EBAModStsArb>"
  Bus Selector6/1 -> pedAEBfullFuncImpAborCondCalc/1(EBAModStsArb)  "<EBAModStsArb>"
  Bus Selector3/5 -> JA_vehAEBfullFuncImpAborCondCalc/5(JA_PTW_AEBModStsArb)  "<JA_PTW_AEBModStsArb>"
  Bus Selector3/6 -> JA_vehAEBfullFuncImpAborCondCalc/6(JA_cycAEBModStsArb)  "<JA_cycAEBModStsArb>"
  Bus Selector3/7 -> JA_vehAEBfullFuncImpAborCondCalc/7(JA_pedAEBModStsArb)  "<JA_pedAEBModStsArb>"
  Bus Selector7/4 -> JA_pedAEBfullFuncImpAborCondCalc/4(EBAModStsArb)  "<EBAModStsArb>"
  Bus Selector7/3 -> JA_pedAEBfullFuncImpAborCondCalc/3(pedAEBfullModStsArb)  "<cycAEBfullModStsArb>"
  Bus Selector7/2 -> JA_pedAEBfullFuncImpAborCondCalc/2(cycAEBfullModStsArb)  "<pedAEBfullModStsArb>"
  Bus Selector7/1 -> JA_pedAEBfullFuncImpAborCondCalc/1(AEBfullModStsArb)  "<AEBfullModStsArb>"
  JA_pedAEBfullFuncImpAborCondCalc/1(JA_pedAEBfullFuncImpAborCond) -> JA_pedAEBfullFuncImpAborCond/1  "JA_pedAEBfullFuncImpAbor"
  Bus Selector8/2 -> JA_cycAEBfullFuncImpAborCondCalc/2(cycAEBfullModStsArb)  "<pedAEBfullModStsArb>"
  Bus Selector8/3 -> JA_cycAEBfullFuncImpAborCondCalc/3(pedAEBfullModStsArb)  "<cycAEBfullModStsArb>"
  Bus Selector8/4 -> JA_cycAEBfullFuncImpAborCondCalc/4(EBAModStsArb)  "<EBAModStsArb>"
  Bus Selector8/1 -> JA_cycAEBfullFuncImpAborCondCalc/1(AEBfullModStsArb)  "<AEBfullModStsArb>"
  Bus Selector8/5 -> JA_cycAEBfullFuncImpAborCondCalc/5(JA_pedAEBModStsArb)  "<JA_pedAEBModStsArb>"
  JA_cycAEBfullFuncImpAborCondCalc/1(JA_cycAEBfullFuncImpAborCond) -> JA_cycAEBfullFuncImpAborCond/1  "JA_cycAEBfullFuncImpAbor"
  Bus Creator/1 -> Bus Selector7/1, Bus Selector9/1, Bus Selector6/1, Bus Selector5/1, Bus Selector4/1, Bus Selector8/1, Bus Selector3/1, Bus Selector2/1, Bus Selector1/1, Bus Selector/1
  Bus Selector9/6 -> JA_PTW_AEBfullFuncImpAborCondCalc/6(EBAModStsArb)  "<EBAModStsArb>"
  Bus Selector9/5 -> JA_PTW_AEBfullFuncImpAborCondCalc/5(JA_vehAEBfullModStsArb)  "<JA_vehAEBModStsArb>"
  Bus Selector9/4 -> JA_PTW_AEBfullFuncImpAborCondCalc/4(cycAEBfullModStsArb)  "<cycAEBfullModStsArb>"
  Bus Selector9/3 -> JA_PTW_AEBfullFuncImpAborCondCalc/3(cycJA_PTW_AEBfullModStsArb)  "<cycAEBpartModStsArb>"
  Bus Selector9/2 -> JA_PTW_AEBfullFuncImpAborCondCalc/2(pedAEBfullModStsArb)  "<pedAEBfullModStsArb>"
  Bus Selector9/1 -> JA_PTW_AEBfullFuncImpAborCondCalc/1(AEBfullModStsArb)  "<AEBfullModStsArb>"
  JA_PTW_AEBfullFuncImpAborCondCalc/1(JA_PTW_AEBfullFuncImpAborCond) -> JA_PTW_AEBfullFuncImpAborCond/1  "JA_PTW_AEBfullFuncImpAbor"
  Bus Selector1/3 -> AEBpartFuncImpAborCondCalc/3(JA_pedAEBfullModStsArb)  "<JA_pedAEBModStsArb>"
  Bus Selector2/3 -> cycAEBpartFuncImpAborCondCalc/3(JA_pedAEBfullModStsArb)  "<JA_pedAEBModStsArb>"

== system TosAeb/FcwAebJa_Step_sys/ALG_AEBSystem/ALG_AEBController/AEB_FuncImpManagementApp/FuncImpArbCalc/BrkFuncImpArbCalc/BrkFuncImpArbCalc ==
  AEBModStsArb : Inport
  brkFuncImpAborCondCond : Inport  Port=2
  FCTBModStsArb : Inport  Port=3
  AEBfullFuncImpArbCalc : Subsystem
  AEBpartFuncImpArbCalc : Subsystem
  Bus Creator : BusCreator  Inputs=2
  Bus Selector : BusSelector  OutputSignals=AEBModStsArb.AEBpartModStsArb,BrkFuncImpAborCond.vehAEBpartFuncImpAborCond
  Bus Selector1 : BusSelector  OutputSignals=AEBModStsArb.cycAEBpartModStsArb,BrkFuncImpAborCond.cycAEBpartFuncImpAborCond
  Bus Selector10 : BusSelector  OutputSignals=AEBModStsArb.JA_pedAEBModStsArb,BrkFuncImpAborCond.JA_pedAEBfullFuncImpAborCond
  Bus Selector2 : BusSelector  OutputSignals=AEBModStsArb.AEBfullModStsArb,BrkFuncImpAborCond.vehAEBfullFuncImpAborCond
  Bus Selector3 : BusSelector  OutputSignals=AEBModStsArb.JA_vehAEBModStsArb,BrkFuncImpAborCond.JA_vehAEBfullFuncImpAborCond
  Bus Selector4 : BusSelector  OutputSignals=AEBModStsArb.cycAEBfullModStsArb,BrkFuncImpAborCond.cycAEBfullFuncImpAborCond
  Bus Selector5 : BusSelector  OutputSignals=AEBModStsArb.pedAEBfullModStsArb,BrkFuncImpAborCond.pedAEBfullFuncImpAborCond
  Bus Selector6 : BusSelector  OutputSignals=BrkFuncImpAborCond.FCTBFuncImpAborCond
  Bus Selector7 : BusSelector  OutputSignals=AEBModStsArb.EBAModStsArb,BrkFuncImpAborCond.vehEBAFuncImpAborCond
  Bus Selector8 : BusSelector  OutputSignals=AEBModStsArb.JA_PTW_AEBModStsArb,BrkFuncImpAborCond.JA_PTW_AEBfullFuncImpAborCond
  Bus Selector9 : BusSelector  OutputSignals=AEBModStsArb.JA_cycAEBModStsArb,BrkFuncImpAborCond.JA_cycAEBfullFuncImpAborCond
  EBAFuncImpArbCalc : Subsystem
  FCTBFuncImpArbCalc : Subsystem
  JA_PTW_AEBFuncImpArbCalc : Subsystem
  JA_cycAEBFuncImpArbCalc : Subsystem
  JA_pedAEBFuncImpArbCalc3 : Subsystem
  JA_vehAEBfullFuncImpArbCalc : Subsystem
  cycAEBfullFuncImpArbCalc : Subsystem
  cycAEBpartFuncImpArbCalc : Subsystem
  pedAEBfullFuncImpArbCalc : Subsystem
  AEBpartFuncImpArb : Outport
  cycAEBpartFuncImpArb : Outport  Port=2
  AEBfullFuncImpArb : Outport  Port=3
  JA_vehAEBfullFuncImpArb : Outport  Port=4
  cycAEBfullFuncImpArb : Outport  Port=5
  pedAEBfullFuncImpArb : Outport  Port=6
  EBAFuncImpArb : Outport  Port=7
  FCTBFuncImpArb : Outport  Port=8
  JA_PTW_AEBFuncImpArb : Outport  Port=9
  JA_cycAEBFuncImpArb : Outport  Port=10
  JA_pedAEBFuncImpArb : Outport  Port=11
  wires:
  Bus Selector10/2 -> JA_pedAEBFuncImpArbCalc3/2(JA_pedAEBFuncImpAborCond)  "<JA_pedAEBfullFuncImpAborCond>"
  Bus Selector10/1 -> JA_pedAEBFuncImpArbCalc3/1(JA_pedAEBModStsArb)  "<JA_pedAEBModStsArb>"
  JA_pedAEBFuncImpArbCalc3/1(JA_pedAEBFuncImpArb) -> JA_pedAEBFuncImpArb/1  "JA_pedAEBFuncImpArb"
  Bus Creator/1 -> Bus Selector7/1, Bus Selector6/1, Bus Selector10/1, Bus Selector9/1, Bus Selector8/1, Bus Selector5/1, Bus Selector4/1, Bus Selector3/1, Bus Selector2/1, Bus Selector1/1, Bus Selector/1
  JA_cycAEBFuncImpArbCalc/1(JA_cycAEBFuncImpArb) -> JA_cycAEBFuncImpArb/1  "JA_cycAEBFuncImpArb"
  Bus Selector9/1 -> JA_cycAEBFuncImpArbCalc/1(JA_cycAEBModStsArb)  "<JA_cycAEBModStsArb>"
  Bus Selector9/2 -> JA_cycAEBFuncImpArbCalc/2(JA_cycAEBFuncImpAborCond)  "<JA_cycAEBfullFuncImpAborCond>"
  Bus Selector8/2 -> JA_PTW_AEBFuncImpArbCalc/2(JA_PTW_AEBFuncImpAborCond)  "<JA_PTW_AEBfullFuncImpAborCond>"
  Bus Selector8/1 -> JA_PTW_AEBFuncImpArbCalc/1(JA_PTW_AEBModStsArb)  "<JA_PTW_AEBModStsArb>"
  JA_PTW_AEBFuncImpArbCalc/1(JA_PTW_AEBFuncImpArb) -> JA_PTW_AEBFuncImpArb/1  "JA_PTW_AEBFuncImpArb"
  FCTBFuncImpArbCalc/1(FCTBFuncImpArb) -> FCTBFuncImpArb/1  "FCTBFuncImpArb"
  EBAFuncImpArbCalc/1(EBAFuncImpArb) -> EBAFuncImpArb/1  "EBAFuncImpArb"
  pedAEBfullFuncImpArbCalc/1(pedAEBfullFuncImpArb) -> pedAEBfullFuncImpArb/1  "pedAEBfullFuncImpArb"
  cycAEBfullFuncImpArbCalc/1(cycAEBfullFuncImpArb) -> cycAEBfullFuncImpArb/1  "cycAEBfullFuncImpArb"
  JA_vehAEBfullFuncImpArbCalc/1(JA_vehAEBfullFuncImpArb) -> JA_vehAEBfullFuncImpArb/1  "JA_vehAEBfullFuncImpArb"
  AEBfullFuncImpArbCalc/1(AEBfullFuncImpArb) -> AEBfullFuncImpArb/1  "AEBfullFuncImpArb"
  cycAEBpartFuncImpArbCalc/1(cycAEBpartFuncImpArb) -> cycAEBpartFuncImpArb/1  "cycAEBpartFuncImpArb"
  Bus Selector6/1 -> FCTBFuncImpArbCalc/2(FCTBFuncImpAborCond)  "<FCTBFuncImpAborCond>"
  FCTBModStsArb/1 -> FCTBFuncImpArbCalc/1(FCTBModStsArb)
  Bus Selector7/1 -> EBAFuncImpArbCalc/1(EBAModStsArb)  "<EBAModStsArb>"
  Bus Selector7/2 -> EBAFuncImpArbCalc/2(EBAFuncImpAborCond)  "<vehEBAFuncImpAborCond>"
  Bus Selector2/1 -> AEBfullFuncImpArbCalc/1(AEBfullModStsArb)  "<AEBfullModStsArb>"
  Bus Selector3/1 -> JA_vehAEBfullFuncImpArbCalc/1(JA_vehAEBfullfullModStsArb)  "<JA_vehAEBModStsArb>"
  Bus Selector4/1 -> cycAEBfullFuncImpArbCalc/1(cycAEBfullModStsArb)  "<cycAEBfullModStsArb>"
  Bus Selector5/1 -> pedAEBfullFuncImpArbCalc/1(pedAEBfullModStsArb)  "<pedAEBfullModStsArb>"
  Bus Selector5/2 -> pedAEBfullFuncImpArbCalc/2(pedAEBfullFuncImpAborCond)  "<pedAEBfullFuncImpAborCond>"
  Bus Selector4/2 -> cycAEBfullFuncImpArbCalc/2(cycAEBfullFuncImpAborCond)  "<cycAEBfullFuncImpAborCond>"
  Bus Selector3/2 -> JA_vehAEBfullFuncImpArbCalc/2(JA_vehAEBfullFuncImpAborCond)  "<JA_vehAEBfullFuncImpAborCond>"
  Bus Selector2/2 -> AEBfullFuncImpArbCalc/2(AEBfullFuncImpAborCond)  "<vehAEBfullFuncImpAborCond>"
  Bus Selector1/1 -> cycAEBpartFuncImpArbCalc/1(cycAEBpartModStsArb)  "<cycAEBpartModStsArb>"
  Bus Selector1/2 -> cycAEBpartFuncImpArbCalc/2(cycAEBpartFuncImpAborCond)  "<cycAEBpartFuncImpAborCond>"
  AEBpartFuncImpArbCalc/1(AEBpartFuncImpArb) -> AEBpartFuncImpArb/1  "AEBpartFuncImpArb"
  Bus Selector/2 -> AEBpartFuncImpArbCalc/2(AEBpartFuncImpAborCond)  "<vehAEBpartFuncImpAborCond>"
  Bus Selector/1 -> AEBpartFuncImpArbCalc/1(AEBpartModStsArb)  "<AEBpartModStsArb>"
  brkFuncImpAborCondCond/1 -> Bus Creator/2  "BrkFuncImpAborCond"
  AEBModStsArb/1 -> Bus Creator/1  "AEBModStsArb"
